simulink model "ofdm_txrx_supermimo_coded"  (Simulink 7.7)
settings: Solver=VariableStepDiscrete  SolverMode=SingleTasking  StartTime=0.0  StopTime=2*simOnly_simLength  RelTol=1e-3
PostLoadFcn:
  | ofdm_rx_supermimo_init
InitFcn:
  | ofdm_rx_supermimo_init
StartFcn:
  | ofdm_rx_supermimo_init
values from neighbouring files (symbols this model uses):
  ActionID_AFTransmit = 31    [ofdm_tx_supermimo_init.m]
  ActionID_Disabled = 0    [ofdm_tx_supermimo_init.m]
  ActionID_SetFlagA = 62    [ofdm_tx_supermimo_init.m]
  ActionID_SetFlagB = 61    [ofdm_tx_supermimo_init.m]
  BER_RAM_init_size = 2048    [ofdm_tx_supermimo_init.m]
  channel_h = [1 0; 1 0] * 0.3    [ofdm_tx_supermimo_init.m]
  CPLength = 16    [ofdm_tx_supermimo_init.m]
  longCorr_coef_i = [-4*real(LTS) 0]    [ofdm_rx_supermimo_init.m]
  longCorr_coef_q = [4*imag(LTS) 0]    [ofdm_rx_supermimo_init.m]
  max_CP_length = 16    [ofdm_tx_supermimo_init.m]
  max_num_baseRateSymbols = 31    [ofdm_tx_supermimo_init.m]
  max_num_subcarriers = 64    [ofdm_tx_supermimo_init.m]
  max_num_trainingSymbols = 15    [ofdm_tx_supermimo_init.m]
  max_numBytes = 16384    [ofdm_tx_supermimo_init.m]
  max_OFDM_symbols = 1023    [ofdm_tx_supermimo_init.m]
  modConstellation_qam16 = .75*[1 3 -1 -3]./3    [ofdm_tx_supermimo_init.m]
  modConstellation_qam64 = 0.875*[3 1 5 7 -3 -1 -5 -7]./7    [ofdm_tx_supermimo_init.m]
  modConstellation_qpsk = [1 -1]./sqrt(2)    [ofdm_tx_supermimo_init.m]
  numBaseRateSymbols = numBaseRateSymbols *2    [ofdm_tx_supermimo_init.m]
  numSubcarriers = 64    [ofdm_tx_supermimo_init.m]
  numTrainingSymbols = 2    [ofdm_tx_supermimo_init.m]
  pktTiming_count_payloadStart = pktTiming_count_lsStart + pktTiming_ls_num*pktTiming_ls_length    [ofdm_rx_supermimo_init.m]
  preamble = preamble_scale*[shortsyms_10 longsyms_2]    [ofdm_tx_supermimo_init.m]
  preamble_ROM = preamble_I_ROM + preamble_Q_ROM*2^16    [ofdm_tx_supermimo_init.m]
  RAM_init_size = max_numBytes    (= 16384)    [ofdm_tx_supermimo_init.m]
  RxDataScrambling_Seq = [40 198 78 63 82 173 102 245 48 111 172 115 147 230 216 93 72 65 62 2 205 242 122 90 128 83 105 97 73 10 5 252]    [ofdm_tx_supermimo_init.m]
  shortSymbol_time = shortSymbol_time(1:16).'    [ofdm_tx_supermimo_init.m]
  simOnly_numSamples = length(preamble)+( (numSubcarriers+CPLength)*(numTrainingSymbols + numBaseRateSymbols + numFullRateSymbols/2) )    [ofdm_tx_supermimo_init.m]
  train = [train train]    [ofdm_tx_supermimo_init.m]
  TxDataScrambling_Seq = [40 198 78 63 82 173 102 245 48 111 172 115 147 230 216 93 72 65 62 2 205 242 122 90 128 83 105 97 73 10 5 252]    [ofdm_tx_supermimo_init.m]
undefined symbols (not defined in the file or in its folder): AntA, AntB, BaseRate, Down, gcbh, numBuffs, numBytes, Up, xlBlockLoadCallback, xlGetNormalizedPeriod, xlipgetpartsetting
libraries: xbsIndex_r4 x5430
(part 18 of 49)

== system ofdm_txrx_supermimo_coded/OFDM Rx MIMO/Equalizer & Packetizer/Packet_Constructor/Slicer ==
  note: 8MSB
  note: 8MSB-7
  SliceSel : Inport
  D : Inport  Port=2
  " byte mux1" : xbsIndex_r4/Mux  inputs=8  en=off  latency=0  precision=Full  arith_type="Signed  (2's comp)"  n_bits=8  bin_pt=2  quantization=Truncate  overflow=Wrap  dbl_ovrd=off  block_type=mux
      settings: xl_area=[16 0 0 32 0 0 0]  block_version=VER_STRING_GOES_HERE
  Slice : xbsIndex_r4/Slice  nbits=8  boolean_output=off  mode=Lower Bit Location + Width  bit1=0  base1=MSB of Input  bit0=0  base0=LSB of Input  dbl_ovrd=off  block_type=slice
      settings: infoedit="Extracts a given range of bits from each input sample and presents it at the output.  The output type is ordinarily unsigned with binary point at zero, but can be Boolean when the slice is one bit wide.<P><P>Hardware notes: In hardware this block costs nothing."  block_version=VER_STRING_GOES_HERE
  Slice1 : xbsIndex_r4/Slice  nbits=8  boolean_output=off  mode=Lower Bit Location + Width  bit1=0  base1=MSB of Input  bit0=1  base0=LSB of Input  dbl_ovrd=off  block_type=slice
      settings: infoedit="Extracts a given range of bits from each input sample and presents it at the output.  The output type is ordinarily unsigned with binary point at zero, but can be Boolean when the slice is one bit wide.<P><P>Hardware notes: In hardware this block costs nothing."  block_version=VER_STRING_GOES_HERE
  Slice2 : xbsIndex_r4/Slice  nbits=8  boolean_output=off  mode=Lower Bit Location + Width  bit1=0  base1=MSB of Input  bit0=2  base0=LSB of Input  dbl_ovrd=off  block_type=slice
      settings: infoedit="Extracts a given range of bits from each input sample and presents it at the output.  The output type is ordinarily unsigned with binary point at zero, but can be Boolean when the slice is one bit wide.<P><P>Hardware notes: In hardware this block costs nothing."  block_version=VER_STRING_GOES_HERE
  Slice3 : xbsIndex_r4/Slice  nbits=8  boolean_output=off  mode=Lower Bit Location + Width  bit1=0  base1=MSB of Input  bit0=3  base0=LSB of Input  dbl_ovrd=off  block_type=slice
      settings: infoedit="Extracts a given range of bits from each input sample and presents it at the output.  The output type is ordinarily unsigned with binary point at zero, but can be Boolean when the slice is one bit wide.<P><P>Hardware notes: In hardware this block costs nothing."  block_version=VER_STRING_GOES_HERE
  Slice4 : xbsIndex_r4/Slice  nbits=8  boolean_output=off  mode=Lower Bit Location + Width  bit1=0  base1=MSB of Input  bit0=4  base0=LSB of Input  dbl_ovrd=off  block_type=slice
      settings: infoedit="Extracts a given range of bits from each input sample and presents it at the output.  The output type is ordinarily unsigned with binary point at zero, but can be Boolean when the slice is one bit wide.<P><P>Hardware notes: In hardware this block costs nothing."  block_version=VER_STRING_GOES_HERE
  Slice5 : xbsIndex_r4/Slice  nbits=8  boolean_output=off  mode=Lower Bit Location + Width  bit1=0  base1=MSB of Input  bit0=5  base0=LSB of Input  dbl_ovrd=off  block_type=slice
      settings: infoedit="Extracts a given range of bits from each input sample and presents it at the output.  The output type is ordinarily unsigned with binary point at zero, but can be Boolean when the slice is one bit wide.<P><P>Hardware notes: In hardware this block costs nothing."  block_version=VER_STRING_GOES_HERE
  Slice6 : xbsIndex_r4/Slice  nbits=8  boolean_output=off  mode=Lower Bit Location + Width  bit1=0  base1=MSB of Input  bit0=6  base0=LSB of Input  dbl_ovrd=off  block_type=slice
      settings: infoedit="Extracts a given range of bits from each input sample and presents it at the output.  The output type is ordinarily unsigned with binary point at zero, but can be Boolean when the slice is one bit wide.<P><P>Hardware notes: In hardware this block costs nothing."  block_version=VER_STRING_GOES_HERE
  Slice7 : xbsIndex_r4/Slice  nbits=8  boolean_output=off  mode=Lower Bit Location + Width  bit1=0  base1=MSB of Input  bit0=7  base0=LSB of Input  dbl_ovrd=off  block_type=slice
      settings: infoedit="Extracts a given range of bits from each input sample and presents it at the output.  The output type is ordinarily unsigned with binary point at zero, but can be Boolean when the slice is one bit wide.<P><P>Hardware notes: In hardware this block costs nothing."  block_version=VER_STRING_GOES_HERE
  Q : Outport
  wires:
  " byte mux1"/1 -> Q/1
  SliceSel/1 -> " byte mux1"/1
  D/1 -> Slice3/1, Slice6/1, Slice7/1, Slice5/1, Slice4/1, Slice2/1, Slice1/1, Slice/1
  Slice/1 -> " byte mux1"/2
  Slice1/1 -> " byte mux1"/3
  Slice2/1 -> " byte mux1"/4
  Slice3/1 -> " byte mux1"/5
  Slice4/1 -> " byte mux1"/6
  Slice5/1 -> " byte mux1"/7
  Slice6/1 -> " byte mux1"/8
  Slice7/1 -> " byte mux1"/9

== system ofdm_txrx_supermimo_coded/OFDM Rx MIMO/Equalizer & Packetizer/Packet_Constructor/Subsystem ==
  In1 : Inport
  In2 : Inport  Port=2
  In3 : Inport  Port=3
  In4 : Inport  Port=4
  In5 : Inport  Port=5
  In6 : Inport  Port=6
  In7 : Inport  Port=7
  In8 : Inport  Port=8
  Simulation Multiplexer{,1..7} (8 alike) : xbsIndex_r4/Simulation Multiplexer  sim_sel=1  hw_sel=2  block_type=simmux
      settings: infoedit="Distinguish input subsystems as \"simulation only\" and \"generation only\".  The input specified For Simulation will be used during Simulink simulation.  The input specified For Generation will be used during code generation.  This block will typically be used on the output of a HW Co-Simulation, Black Box, or ModelSim subsystem.<br><br>Hardware Notes: This block costs nothing."
  Subsystem : Subsystem
  Subsystem1 : Subsystem
  Out1 : Outport
  Out2 : Outport  Port=2
  Out3 : Outport  Port=3
  Out4 : Outport  Port=4
  Out5 : Outport  Port=5
  Out6 : Outport  Port=6
  Out7 : Outport  Port=7
  Out8 : Outport  Port=8
  wires:
  In1/1 -> Subsystem/1(In1), Subsystem1/1(In1)
  In3/1 -> Subsystem/3(In3), Subsystem1/3(In3)
  In4/1 -> Subsystem/4(In4), Subsystem1/4(In4)
  In5/1 -> Subsystem/5(In5), Subsystem1/5(In5)
  In6/1 -> Subsystem/6(In6), Subsystem1/6(In6)
  In7/1 -> Subsystem/7(In7), Subsystem1/7(In7)
  In8/1 -> Subsystem/8(In8), Subsystem1/8(In8)
  In2/1 -> Subsystem/2(In2), Subsystem1/2(In2)
  Simulation Multiplexer/1 -> Out1/1
  Simulation Multiplexer1/1 -> Out2/1
  Simulation Multiplexer2/1 -> Out3/1
  Simulation Multiplexer3/1 -> Out4/1
  Simulation Multiplexer4/1 -> Out5/1
  Simulation Multiplexer5/1 -> Out6/1
  Simulation Multiplexer6/1 -> Out7/1
  Simulation Multiplexer7/1 -> Out8/1
  Subsystem/1(Out1) -> Simulation Multiplexer/1
  Subsystem/2(Out2) -> Simulation Multiplexer1/1
  Subsystem/3(Out3) -> Simulation Multiplexer2/1
  Subsystem/4(Out4) -> Simulation Multiplexer3/1
  Subsystem/5(Out5) -> Simulation Multiplexer4/1
  Subsystem/6(Out6) -> Simulation Multiplexer5/1
  Subsystem/7(Out7) -> Simulation Multiplexer6/1
  Subsystem/8(Out8) -> Simulation Multiplexer7/1
  Subsystem1/1(Out1) -> Simulation Multiplexer/2
  Subsystem1/2(Out2) -> Simulation Multiplexer1/2
  Subsystem1/3(Out3) -> Simulation Multiplexer2/2
  Subsystem1/4(Out4) -> Simulation Multiplexer3/2
  Subsystem1/5(Out5) -> Simulation Multiplexer4/2
  Subsystem1/6(Out6) -> Simulation Multiplexer5/2
  Subsystem1/7(Out7) -> Simulation Multiplexer6/2
  Subsystem1/8(Out8) -> Simulation Multiplexer7/2

== system ofdm_txrx_supermimo_coded/OFDM Rx MIMO/FFT & Chan Est/Addr Gen1/Copy_Downsample ==
  D : Inport
  Delay : xbsIndex_r4/Delay  rst=off  infoeditControl=Selection of Reset will increase slice count due to use of real FFs and instead of SRLs  en=off  latency=1  dbl_ovrd=off  reg_retiming=off  block_type=delay
      settings: infoedit=Hardware notes: A delay line is a chain, each link of which is an SRL16 followed by a flip-flop. If register retiming is enabled, the delay line is a chain of flip-flops.  block_version=VER_STRING_GOES_HERE
  Down Sample : xbsIndex_r4/Down Sample  sample_ratio=2  sample_phase="Last Value of Frame  (most efficient)"  en=off  latency=1  dbl_ovrd=off  block_type=dsamp
      settings: block_version=VER_STRING_GOES_HERE
  Logical : xbsIndex_r4/Logical  logical_function=OR  inputs=2  en=off  latency=0  precision=Full  arith_type=Unsigned  n_bits=16  bin_pt=0  align_bp=on  dbl_ovrd=off  block_type=logical
      settings: block_version=VER_STRING_GOES_HERE
  Q : Outport
  wires:
  Delay/1 -> Logical/1
  D/1 -> Delay/1, Logical/2
  Logical/1 -> Down Sample/1
  Down Sample/1 -> Q/1

== system ofdm_txrx_supermimo_coded/OFDM Rx MIMO/FFT & Chan Est/Addr Gen1/Oscillator ==
  en : Inport
  rst : Inport  Port=2
  Assert : xbsIndex_r4/Assert  assert_type=on  type_source=Explicitly  gui_display_data_type=Fixed-point  arith_type=Boolean  n_bits=16  bin_pt=14  preci_type=Single  exp_width=8  frac_width=24  assert_rate=on  rate_source=Explicitly  period=2  output_port=on  block_type=assert
      settings: infoedit=Asserts a user-defined sample rate and/or type on a signal.<P><P>Hardware notes: In hardware this block costs nothing.  block_version=VER_STRING_GOES_HERE
  Inverter1 : xbsIndex_r4/Inverter  en=off  latency=0  dbl_ovrd=off  block_type=inv
      settings: block_version=VER_STRING_GOES_HERE
  Register : xbsIndex_r4/Register  init=0  rst=on  en=on  dbl_ovrd=off  block_type=register
      settings: block_version=VER_STRING_GOES_HERE
  Q : Outport
  wires:
  Register/1 -> Q/1, Assert/1
  en/1 -> Register/3
  Inverter1/1 -> Register/1
  rst/1 -> Register/2
  Assert/1 -> Inverter1/1

== system ofdm_txrx_supermimo_coded/OFDM Rx MIMO/FFT & Chan Est/Addr Gen1/Output Sym Counter ==
  FFT Done : Inport
  Not Training : Inport  Port=2
  Payload : Inport  Port=3
  Read Addr : Inport  Port=4
  Constant1 : xbsIndex_r4/Constant  const=numSubcarriers-1  gui_display_data_type=Fixed-point  arith_type=Unsigned  n_bits=ceil(log2(numSubcarriers))  bin_pt=0  preci_type=Single  exp_width=8  frac_width=24  explicit_period=off  period=1  equ=P=C  opselect=C  inp2=PCIN>>17  opr=+  inp1=P  carry=CIN  dbl_ovrd=off  block_type=constant
      settings: block_version=VER_STRING_GOES_HERE
  Counter1 : xbsIndex_r4/Counter  cnt_type=Free Running  cnt_to=127  operation=Up  start_count=0  cnt_by_val=1  arith_type=Unsigned  n_bits=ceil(log2(max_OFDM_symbols))  bin_pt=0  load_pin=off  rst=on  en=on  explicit_period=off  period=2  dbl_ovrd=off  use_behavioral_HDL=off  implementation=Fabric  use_rpm=on  block_type=counter
      settings: xl_area=[6 12 0 12 0 0 0]  block_version=VER_STRING_GOES_HERE
  Inverter3 : xbsIndex_r4/Inverter  en=off  latency=0  dbl_ovrd=off  block_type=inv
      settings: block_version=VER_STRING_GOES_HERE
  Logical1 : xbsIndex_r4/Logical  logical_function=AND  inputs=2  en=off  latency=0  precision=Full  arith_type=Unsigned  n_bits=8  bin_pt=2  align_bp=on  dbl_ovrd=off  block_type=logical
      settings: xl_area=[0, 0, 0, 0, 0, 0, 0]  block_version=VER_STRING_GOES_HERE
  Logical2 : xbsIndex_r4/Logical  logical_function=OR  inputs=2  en=off  latency=0  precision=Full  arith_type=Unsigned  n_bits=8  bin_pt=2  align_bp=on  dbl_ovrd=off  block_type=logical
      settings: xl_area=[0, 0, 0, 0, 0, 0, 0]  block_version=VER_STRING_GOES_HERE
  Logical3 : xbsIndex_r4/Logical  logical_function=AND  inputs=2  en=off  latency=0  precision=Full  arith_type=Unsigned  n_bits=8  bin_pt=2  align_bp=on  dbl_ovrd=off  block_type=logical
      settings: xl_area=[0, 0, 0, 0, 0, 0, 0]  block_version=VER_STRING_GOES_HERE
  Logical4 : xbsIndex_r4/Logical  logical_function=AND  inputs=2  en=off  latency=0  precision=Full  arith_type=Unsigned  n_bits=8  bin_pt=2  align_bp=on  dbl_ovrd=off  block_type=logical
      settings: xl_area=[0, 0, 0, 0, 0, 0, 0]  block_version=VER_STRING_GOES_HERE
  Posedge : Subsystem
  Posedge1 : Subsystem
  Posedge2 : Subsystem
  Posedge3 : Subsystem
  Relational : xbsIndex_r4/Relational  mode=a=b  en=off  latency=0  dbl_ovrd=off  block_type=relational
      settings: block_version=VER_STRING_GOES_HERE
  S-R Latch2 : Subsystem
  Sym Index : Outport
  wires:
  Logical4/1 -> Logical3/2
  S-R Latch2/1(D) -> Logical4/2
  Posedge/1(Q) -> S-R Latch2/1(S), Logical4/1
  FFT Done/1 -> Posedge/1(D)
  Counter1/1 -> Sym Index/1
  Not Training/1 -> Inverter3/1, Logical1/2
  Inverter3/1 -> Logical3/1
  Payload/1 -> Posedge1/1(D)
  Posedge1/1(Q) -> S-R Latch2/2(R), Counter1/1
  Read Addr/1 -> Relational/2
  Constant1/1 -> Relational/1
  Relational/1 -> Logical1/1
  Logical1/1 -> Posedge2/1(D)
  Logical3/1 -> Posedge3/1(D)
  Logical2/1 -> Counter1/2
  Posedge2/1(Q) -> Logical2/1
  Posedge3/1(Q) -> Logical2/2

== system ofdm_txrx_supermimo_coded/OFDM Rx MIMO/FFT & Chan Est/Addr Gen1/Rd Delay ==
  note: Delay the equalizer inputs in Alamout mode in order
      | to calculate pilot phaes for both r0 and r1 before
      | starting the decoding process. In SISO/Multiplexing mode,
      | each symbol has its own pilots, so there's a shorter delay.
  D : Inport
  Delay1 : xbsIndex_r4/Delay  rst=off  infoeditControl=Selection of Reset will increase slice count due to use of real FFs and instead of SRLs  en=off  latency=3  dbl_ovrd=off  reg_retiming=off  block_type=delay
      settings: block_version=10.1.2
  Delay2 : xbsIndex_r4/Delay  rst=off  infoeditControl=Selection of Reset will increase slice count due to use of real FFs and instead of SRLs  en=off  latency=35  dbl_ovrd=off  reg_retiming=off  block_type=delay
      settings: block_version=10.1.2
  Inverter1 : xbsIndex_r4/Inverter  en=off  latency=0  dbl_ovrd=off  block_type=inv
  Logical1 : xbsIndex_r4/Logical  logical_function=AND  inputs=2  en=off  latency=0  precision=Full  arith_type=Unsigned  n_bits=16  bin_pt=0  align_bp=on  dbl_ovrd=off  block_type=logical
  Logical2 : xbsIndex_r4/Logical  logical_function=OR  inputs=2  en=off  latency=0  precision=Full  arith_type=Unsigned  n_bits=16  bin_pt=0  align_bp=on  dbl_ovrd=off  block_type=logical
  Logical3 : xbsIndex_r4/Logical  logical_function=AND  inputs=2  en=off  latency=0  precision=Full  arith_type=Unsigned  n_bits=16  bin_pt=0  align_bp=on  dbl_ovrd=off  block_type=logical
  Q : Outport
  wires:
  Logical1/1 -> Logical2/1
  Logical3/1 -> Logical2/2
  Logical2/1 -> Q/1
  [regRx_AlamoutiMode] -> Logical1/2, Inverter1/1
  Inverter1/1 -> Logical3/1
  Delay2/1 -> Logical1/1
  D/1 -> Delay2/1, Delay1/1
  Delay1/1 -> Logical3/2

== system ofdm_txrx_supermimo_coded/OFDM Rx MIMO/FFT & Chan Est/Addr Gen1/S-R Latch1 ==
  S : Inport
  R : Inport  Port=2
  Constant2 : xbsIndex_r4/Constant  const=1  gui_display_data_type=Fixed-point  arith_type=Boolean  n_bits=1  bin_pt=0  preci_type=Single  exp_width=8  frac_width=24  explicit_period=off  period=1  equ=P=C  opselect=C  inp2=PCIN>>17  opr=+  inp1=P  carry=CIN  dbl_ovrd=off  block_type=constant
      settings: block_version=VER_STRING_GOES_HERE
  Register : xbsIndex_r4/Register  init=0  rst=on  en=on  dbl_ovrd=off  block_type=register
      settings: xl_area=[0, 0, 0, 0, 0, 0, 0]  block_version=VER_STRING_GOES_HERE
  D : Outport
  wires:
  Register/1 -> D/1
  S/1 -> Register/3
  R/1 -> Register/2
  Constant2/1 -> Register/1

== system ofdm_txrx_supermimo_coded/OFDM Rx MIMO/FFT & Chan Est/Addr_Generator/Initial Read Addr Timing Calc ==
  note: The FFT runs 4x as fast as the input samples. As the input
      | samples are written to the buffers, the FFT waits to start
      | until it can have access to the newest sample it needs
      | 1 cycle after it's written.
  AddSub1 : xbsIndex_r4/AddSub  mode=Addition  use_carryin=off  use_carryout=off  en=off  latency=0  precision=User Defined  arith_type=Unsigned  n_bits=ceil(log2(max_num_subcarriers))+1  bin_pt=0  quantization=Truncate  overflow=Wrap  dbl_ovrd=off  use_behavioral_HDL=on  hw_selection=Fabric  pipelined=off  use_rpm=on  block_type=addsub
      settings: xl_area=[4 0 0 6 0 0 0]  block_version=VER_STRING_GOES_HERE
  Constant1 : xbsIndex_r4/Constant  const=0.75*numSubcarriers  gui_display_data_type=Fixed-point  arith_type=Unsigned  n_bits=log2(numSubcarriers)  bin_pt=0  preci_type=Single  exp_width=8  frac_width=24  explicit_period=off  period=1  equ=P=C  opselect=C  inp2=PCIN>>17  opr=+  inp1=P  carry=CIN  dbl_ovrd=off  block_type=constant
      settings: block_version=VER_STRING_GOES_HERE
  Up Sample4 : xbsIndex_r4/Up Sample  sample_ratio=2  copy_samples=on  en=off  latency=0  dbl_ovrd=off  block_type=usamp
      settings: infoedit="Up samples input data.  Inserted values  can be zeros or copies of the most recent input sample.<P><P>Hardware notes: No hardware is needed if inserted values are copies of the input sample; otherwise, a mux and single bit flip-flop are used."  block_version=VER_STRING_GOES_HERE
  D : Outport
  wires:
  AddSub1/1 -> D/1
  [regRx_symbolTimingOffset] -> Up Sample4/1
  Up Sample4/1 -> AddSub1/2
  Constant1/1 -> AddSub1/1

== system ofdm_txrx_supermimo_coded/OFDM Rx MIMO/FFT & Chan Est/Addr_Generator/Oscillator ==
  en : Inport
  rst : Inport  Port=2
  Assert : xbsIndex_r4/Assert  assert_type=on  type_source=Explicitly  gui_display_data_type=Fixed-point  arith_type=Boolean  n_bits=16  bin_pt=14  preci_type=Single  exp_width=8  frac_width=24  assert_rate=on  rate_source=Explicitly  period=4  output_port=on  block_type=assert
      settings: infoedit=Asserts a user-defined sample rate and/or type on a signal.<P><P>Hardware notes: In hardware this block costs nothing.  block_version=VER_STRING_GOES_HERE
  Inverter1 : xbsIndex_r4/Inverter  en=off  latency=0  dbl_ovrd=off  block_type=inv
      settings: block_version=VER_STRING_GOES_HERE
  Register1 : xbsIndex_r4/Register  init=0  rst=on  en=on  dbl_ovrd=off  block_type=register
      settings: block_version=VER_STRING_GOES_HERE
  Q : Outport
  wires:
  rst/1 -> Register1/2
  Assert/1 -> Inverter1/1
  Inverter1/1 -> Register1/1
  en/1 -> Register1/3
  Register1/1 -> Q/1, Assert/1

== system ofdm_txrx_supermimo_coded/OFDM Rx MIMO/FFT & Chan Est/Addr_Generator/Posedge ==
  D : Inport
  Delay : xbsIndex_r4/Delay  rst=off  infoeditControl=Selection of Reset will increase slice count due to use of real FFs and instead of SRLs  en=off  latency=1  dbl_ovrd=off  reg_retiming=off  block_type=delay
      settings: infoedit=Hardware notes: A delay line is a chain, each link of which is an SRL16 followed by a flip-flop. If register retiming is enabled, the delay line is a chain of flip-flops.  xl_area=[1 1 0 0 0 0 0]  block_version=VER_STRING_GOES_HERE
  Inverter : xbsIndex_r4/Inverter  en=off  latency=0  dbl_ovrd=off  block_type=inv
      settings: xl_area=[0 0 0 0 0 0 0]  block_version=VER_STRING_GOES_HERE
  Logical : xbsIndex_r4/Logical  logical_function=AND  inputs=2  en=off  latency=0  precision=Full  arith_type=Unsigned  n_bits=8  bin_pt=2  align_bp=on  dbl_ovrd=off  block_type=logical
      settings: xl_area=[1 0 0 1 0 0 0]  block_version=VER_STRING_GOES_HERE
  Q : Outport
  wires:
  Logical/1 -> Q/1
  D/1 -> Delay/1, Logical/1
  Inverter/1 -> Logical/2
  Delay/1 -> Inverter/1

== system ofdm_txrx_supermimo_coded/OFDM Rx MIMO/FFT & Chan Est/Addr_Generator/negedge ==
  D : Inport
  Delay : xbsIndex_r4/Delay  rst=off  infoeditControl=Selection of Reset will increase slice count due to use of real FFs and instead of SRLs  en=off  latency=1  dbl_ovrd=off  reg_retiming=off  block_type=delay
      settings: infoedit=Hardware notes: A delay line is a chain, each link of which is an SRL16 followed by a flip-flop. If register retiming is enabled, the delay line is a chain of flip-flops.  xl_area=[1 1 0 0 0 0 0]  block_version=VER_STRING_GOES_HERE
  Inverter : xbsIndex_r4/Inverter  en=off  latency=0  dbl_ovrd=off  block_type=inv
      settings: xl_area=[0 0 0 0 0 0 0]  block_version=VER_STRING_GOES_HERE
  Logical : xbsIndex_r4/Logical  logical_function=AND  inputs=2  en=off  latency=0  precision=Full  arith_type=Unsigned  n_bits=8  bin_pt=2  align_bp=on  dbl_ovrd=off  block_type=logical
      settings: xl_area=[1 0 0 1 0 0 0]  block_version=VER_STRING_GOES_HERE
  Q : Outport
  wires:
  Inverter/1 -> Logical/2
  D/1 -> Delay/1, Inverter/1
  Logical/1 -> Q/1
  Delay/1 -> Logical/1

== system ofdm_txrx_supermimo_coded/OFDM Rx MIMO/FFT & Chan Est/Addr_Generator/negedge1 ==
  D : Inport
  Delay : xbsIndex_r4/Delay  rst=off  infoeditControl=Selection of Reset will increase slice count due to use of real FFs and instead of SRLs  en=off  latency=1  dbl_ovrd=off  reg_retiming=off  block_type=delay
      settings: infoedit=Hardware notes: A delay line is a chain, each link of which is an SRL16 followed by a flip-flop. If register retiming is enabled, the delay line is a chain of flip-flops.  xl_area=[1 1 0 0 0 0 0]  block_version=VER_STRING_GOES_HERE
  Inverter : xbsIndex_r4/Inverter  en=off  latency=0  dbl_ovrd=off  block_type=inv
      settings: xl_area=[0 0 0 0 0 0 0]  block_version=VER_STRING_GOES_HERE
  Logical : xbsIndex_r4/Logical  logical_function=AND  inputs=2  en=off  latency=0  precision=Full  arith_type=Unsigned  n_bits=8  bin_pt=2  align_bp=on  dbl_ovrd=off  block_type=logical
      settings: xl_area=[1 0 0 1 0 0 0]  block_version=VER_STRING_GOES_HERE
  Q : Outport
  wires:
  Inverter/1 -> Logical/1
  Delay/1 -> Logical/2
  D/1 -> Inverter/1, Delay/1
  Logical/1 -> Q/1

== system ofdm_txrx_supermimo_coded/OFDM Rx MIMO/FFT & Chan Est/Channel Estimation & Pilot Processing/Actual Trainig Window ==
  H Vin : Inport
  Train Sym : Inport  Port=2
  Logical2 : xbsIndex_r4/Logical  logical_function=AND  inputs=2  en=off  latency=0  precision=Full  arith_type=Unsigned  n_bits=8  bin_pt=2  align_bp=on  dbl_ovrd=off  block_type=logical
      settings: xl_area=[1 0 0 1 0 0 0]  block_version=VER_STRING_GOES_HERE
  Posedge : Subsystem
  S-R Latch : Subsystem
  negedge : Subsystem
  Train : Outport
  wires:
  S-R Latch/1(D) -> Logical2/1
  Posedge/1(Q) -> S-R Latch/1(S)
  negedge/1(Q) -> S-R Latch/2(R)
  H Vin/1 -> Posedge/1(D)
  Logical2/1 -> Train/1
  Train Sym/1 -> negedge/1(D), Logical2/2

== system ofdm_txrx_supermimo_coded/OFDM Rx MIMO/FFT & Chan Est/Channel Estimation & Pilot Processing/Bus Creator ==
  AA-I : Inport
  AA-Q : Inport  Port=2
  AB-I : Inport  Port=3
  AB-Q : Inport  Port=4
  BA-I : Inport  Port=5
  BA-Q : Inport  Port=6
  BB-I : Inport  Port=7
  BB-Q : Inport  Port=8
  Bus Creator1 : BusCreator
  Bus Creator2 : BusCreator
  Constant1 : xbsIndex_r4/Constant  const=0  gui_display_data_type=Fixed-point  arith_type=Signed (2's comp)  n_bits=18  bin_pt=16  preci_type=Single  exp_width=8  frac_width=24  explicit_period=on  period=2  equ=P=C  opselect=C  inp2=PCIN>>17  opr=+  inp1=P  carry=CIN  dbl_ovrd=off  block_type=constant
      settings: block_version=VER_STRING_GOES_HERE
  Constant2 : xbsIndex_r4/Constant  const=0  gui_display_data_type=Fixed-point  arith_type=Signed (2's comp)  n_bits=18  bin_pt=16  preci_type=Single  exp_width=8  frac_width=24  explicit_period=on  period=2  equ=P=C  opselect=C  inp2=PCIN>>17  opr=+  inp1=P  carry=CIN  dbl_ovrd=off  block_type=constant
      settings: block_version=VER_STRING_GOES_HERE
  I : Outport
  Q : Outport  Port=2
  wires:
  AA-I/1 -> Bus Creator1/1
  AB-I/1 -> Bus Creator1/2
  Bus Creator1/1 -> I/1
  BA-I/1 -> Bus Creator1/3
  BB-I/1 -> Bus Creator1/4
  AA-Q/1 -> Bus Creator2/1
  AB-Q/1 -> Bus Creator2/2
  Bus Creator2/1 -> Q/1
  BA-Q/1 -> Bus Creator2/3
  BB-Q/1 -> Bus Creator2/4

== system ofdm_txrx_supermimo_coded/OFDM Rx MIMO/FFT & Chan Est/Channel Estimation & Pilot Processing/Chan Est Buffer & Mag Calc ==
  note: Stores each channel coefficient as a
      | 32-bit word, combining [I Q] per word.
  AA-I : Inport
  AA-Q : Inport  Port=2
  AB-I : Inport  Port=3
  AB-Q : Inport  Port=4
  BA-I : Inport  Port=5
  BA-Q : Inport  Port=6
  BB-I : Inport  Port=7
  BB-Q : Inport  Port=8
  Est Vin : Inport  Port=9
  SubCar Index : Inport  Port=10
  1MSB : xbsIndex_r4/Slice  nbits=1  boolean_output=on  mode=Upper Bit Location + Width  bit1=0  base1=MSB of Input  bit0=0  base0=LSB of Input  dbl_ovrd=off  block_type=slice
      settings: infoedit="Extracts a given range of bits from each input sample and presents it at the output.  The output type is ordinarily unsigned with binary point at zero, but can be Boolean when the slice is one bit wide.<P><P>Hardware notes: In hardware this block costs nothing."  block_version=VER_STRING_GOES_HERE
  "2x16b -> 32b" : Subsystem
  Concat : xbsIndex_r4/Concat  num_inputs=2  dbl_ovrd=off  block_type=concat
      settings: block_version=10.1.3
  Convert{3..10} (8 alike) : xbsIndex_r4/Convert  gui_display_data_type=Fixed-point  arith_type="Signed  (2's comp)"  n_bits=16  bin_pt=14  float_type=Single  exp_bits=8  fraction_bits=24  quantization=Truncate  overflow=Flag as error  en=off  latency=0  dbl_ovrd=off  pipeline=off  block_type=convert
      settings: xl_area=[0, 0, 0, 0, 0, 0, 0]  block_version=VER_STRING_GOES_HERE
  Counter : xbsIndex_r4/Counter  cnt_type=Free Running  cnt_to=Inf  operation=Up  start_count=0  cnt_by_val=1  arith_type=Unsigned  n_bits=2  bin_pt=0  load_pin=off  rst=on  en=on  explicit_period=off  period=1  dbl_ovrd=off  use_behavioral_HDL=off  implementation=Fabric  use_rpm=on  block_type=counter
      settings: xl_area=[6 12 0 12 0 0 0]  block_version=VER_STRING_GOES_HERE
  Delay{1..5} (5 alike) : xbsIndex_r4/Delay  rst=off  infoeditControl=Selection of Reset will increase slice count due to use of real FFs and instead of SRLs  en=off  latency=1  dbl_ovrd=off  reg_retiming=off  block_type=delay
      settings: infoedit=Hardware notes: A delay line is a chain, each link of which is an SRL16 followed by a flip-flop. If register retiming is enabled, the delay line is a chain of flip-flops.  xl_area=[0, 0, 0, 0, 0, 0, 0]  block_version=VER_STRING_GOES_HERE
  Logical1 : xbsIndex_r4/Logical  logical_function=AND  inputs=2  en=off  latency=0  precision=Full  arith_type=Unsigned  n_bits=8  bin_pt=2  align_bp=on  dbl_ovrd=off  block_type=logical
      settings: xl_area=[1 0 0 1 0 0 0]  block_version=VER_STRING_GOES_HERE
  Logical2 : xbsIndex_r4/Logical  logical_function=AND  inputs=2  en=off  latency=0  precision=Full  arith_type=Unsigned  n_bits=8  bin_pt=2  align_bp=on  dbl_ovrd=off  block_type=logical
      settings: xl_area=[1 0 0 1 0 0 0]  block_version=VER_STRING_GOES_HERE
  Mux1 : xbsIndex_r4/Mux  inputs=4  en=off  latency=1  precision=Full  arith_type="Signed  (2's comp)"  n_bits=8  bin_pt=2  quantization=Truncate  overflow=Wrap  dbl_ovrd=off  block_type=mux
      settings: xl_area=[0, 0, 0, 0, 0, 0, 0]  block_version=VER_STRING_GOES_HERE
  Mux2 : xbsIndex_r4/Mux  inputs=4  en=off  latency=1  precision=Full  arith_type="Signed  (2's comp)"  n_bits=8  bin_pt=2  quantization=Truncate  overflow=Wrap  dbl_ovrd=off  block_type=mux
      settings: xl_area=[0, 0, 0, 0, 0, 0, 0]  block_version=VER_STRING_GOES_HERE
  Negedge : Subsystem
  Scope : Scope  NumInputPorts=6
      settings: YMin=-5~-5~-5~-5~-5~-5  YMax=5~5~5~5~5~5  SaveName=ScopeData6
  Shared Memory : xbsIndex_r4/Shared Memory  shared_memory_name='ChannelEstimates'  depth=numSubcarriers * 4  ownership=Locally Owned and Initialized  initVector=zeros(1,numSubcarriers*4)  en=off  mutex=Unprotected  mode=Read and Write  write_mode=Read After Write  time_out=0  latency=1  explicit_data_type=on  gui_display_data_type=Fixed-point  arith_type=Unsigned  n_bits=32  bin_pt=0  preci_type=Single  implementation=Block RAM  use_rpm=off  block_type=shmem
      settings: AttributesFormatString=<< %<shared_memory_name> >>  block_version=10.1.3
  done{1..5,9} (6 alike) : xbsIndex_r4/Gateway Out  inherit_from_input=off  hdl_port=off  timing_constraint=None  locs_specified=off  LOCs={}  UseAsDAC=off  DACChannel='1'  block_type=gatewayout
      settings: infoedit="Gateway out block.  Converts Xilinx fixed point inputs into ouputs of type Simulink integer, double, or fixed point.<P><P>Hardware notes:  In hardware these blocks become top level output ports or are discarded, depending on how they are configured."  xl_area=[0 0 0 0 1 0 0]  block_version=VER_STRING_GOES_HERE
  edge : Subsystem
  wires:
  SubCar Index/1 -> Delay1/1, 1MSB/1, done1/1
  Est Vin/1 -> edge/1(D), Logical1/1, Logical2/2
  1MSB/1 -> Negedge/1(D)
  Negedge/1(Q) -> Logical1/2
  Logical1/1 -> Counter/2
  edge/1(Q) -> Counter/1
  "2x16b -> 32b"/1("[I Q]") -> Shared Memory/2
  Concat/1 -> Delay2/1
  Counter/1 -> Mux2/1, Mux1/1, done5/1, Concat/1
  AA-I/1 -> Convert3/1
  AB-I/1 -> Convert4/1
  BA-I/1 -> Convert5/1
  BB-I/1 -> Convert6/1
  AA-Q/1 -> Convert7/1
  AB-Q/1 -> Convert8/1
  BA-Q/1 -> Convert9/1
  BB-Q/1 -> Convert10/1
  Convert7/1 -> Mux1/2
  Convert8/1 -> Mux1/3
  Convert9/1 -> Mux1/4
  Convert10/1 -> Mux1/5
  Convert3/1 -> Mux2/2
  Convert4/1 -> Mux2/3
  Convert5/1 -> Mux2/4
  Convert6/1 -> Mux2/5
  Mux2/1 -> "2x16b -> 32b"/1(I), done9/1
  Delay1/1 -> Concat/2
  Mux1/1 -> "2x16b -> 32b"/2(Q)
  Delay2/1 -> Delay3/1
  Delay3/1 -> Shared Memory/1, done2/1
  done9/1 -> Scope/1
  done1/1 -> Scope/2
  done2/1 -> Scope/3
  done3/1 -> Scope/4
  done4/1 -> Scope/5
  done5/1 -> Scope/6
  Logical2/1 -> Delay5/1
  [regRx_chanEst_RecordEn] -> Logical2/1
  Delay4/1 -> Shared Memory/3, done4/1
  Delay5/1 -> Delay4/1
  "2x16b -> 32b"/2(H_Mag) -> done3/1

== system ofdm_txrx_supermimo_coded/OFDM Rx MIMO/FFT & Chan Est/Channel Estimation & Pilot Processing/Downsamplng ==
  AA-I : Inport
  AA-Q : Inport  Port=2
  AB-I : Inport  Port=3
  AB-Q : Inport  Port=4
  BA-I : Inport  Port=5
  BA-Q : Inport  Port=6
  BB-I : Inport  Port=7
  BB-Q : Inport  Port=8
  Down Sample{,1..7} (8 alike) : xbsIndex_r4/Down Sample  sample_ratio=2  sample_phase="Last Value of Frame  (most efficient)"  en=off  latency=1  dbl_ovrd=off  block_type=dsamp
      settings: block_version=10.1.2
  " AA-I" : Outport
  " AA-Q" : Outport  Port=2
  " AB-I" : Outport  Port=3
  " AB-Q" : Outport  Port=4
  " BA-I" : Outport  Port=5
  " BA-Q" : Outport  Port=6
  " BB-I" : Outport  Port=7
  " BB-Q" : Outport  Port=8
  wires:
  AA-I/1 -> Down Sample/1
  AB-I/1 -> Down Sample1/1
  AA-Q/1 -> Down Sample4/1
  BA-I/1 -> Down Sample2/1
  AB-Q/1 -> Down Sample5/1
  BB-I/1 -> Down Sample3/1
  BA-Q/1 -> Down Sample6/1
  BB-Q/1 -> Down Sample7/1
  Down Sample/1 -> " AA-I"/1
  Down Sample1/1 -> " AB-I"/1
  Down Sample2/1 -> " BA-I"/1
  Down Sample3/1 -> " BB-I"/1
  Down Sample4/1 -> " AA-Q"/1
  Down Sample5/1 -> " AB-Q"/1
  Down Sample6/1 -> " BA-Q"/1
  Down Sample7/1 -> " BB-Q"/1

== system ofdm_txrx_supermimo_coded/OFDM Rx MIMO/FFT & Chan Est/Channel Estimation & Pilot Processing/H_A-A Estimate Buffer ==
  I : Inport
  Q : Inport  Port=2
  Est_WE : Inport  Port=3
  Est_Reset : Inport  Port=4
  FFT_Addr : Inport  Port=5
  EQ_Addr : Inport  Port=6
  I Estimate Buffer : Subsystem
  Q Estimate Buffer : Subsystem
  EstI_fast : Outport
  EstQ_fast : Outport  Port=2
  EstI : Outport  Port=3
  EstQ : Outport  Port=4
  wires:
  Est_Reset/1 -> I Estimate Buffer/4(Rst), Q Estimate Buffer/4(Rst)
  Est_WE/1 -> I Estimate Buffer/3(Est_WE), Q Estimate Buffer/3(Est_WE)
  I Estimate Buffer/1(h[n]) -> EstI/1
  FFT_Addr/1 -> I Estimate Buffer/2(Fast_Addr), Q Estimate Buffer/2(Fast_Addr)
  I/1 -> I Estimate Buffer/1(Est_Data)
  I Estimate Buffer/2(h[n] (fast)) -> EstI_fast/1
  EQ_Addr/1 -> I Estimate Buffer/5(Slow_Addr), Q Estimate Buffer/5(Slow_Addr)
  Q/1 -> Q Estimate Buffer/1(Est_Data)
  Q Estimate Buffer/1(h[n]) -> EstQ/1
  Q Estimate Buffer/2(h[n] (fast)) -> EstQ_fast/1

== system ofdm_txrx_supermimo_coded/OFDM Rx MIMO/FFT & Chan Est/Channel Estimation & Pilot Processing/H_A-B Estimate Buffer ==
  I : Inport
  Q : Inport  Port=2
  Est_WE : Inport  Port=3
  Est_Reset : Inport  Port=4
  Xk_Index : Inport  Port=5
  I Estimate Buffer : Subsystem
  Q Estimate Buffer : Subsystem
  EstI : Outport
  EstQ : Outport  Port=2
  wires:
  I/1 -> I Estimate Buffer/1(Est_Data)
  Xk_Index/1 -> I Estimate Buffer/2(Est_Addr), Q Estimate Buffer/2(Est_Addr)
  I Estimate Buffer/1(h[n]) -> EstI/1
  Est_WE/1 -> I Estimate Buffer/3(Est_WE), Q Estimate Buffer/3(Est_WE)
  Est_Reset/1 -> I Estimate Buffer/4(Rst), Q Estimate Buffer/4(Rst)
  Q Estimate Buffer/1(h[n]) -> EstQ/1
  Q/1 -> Q Estimate Buffer/1(Est_Data)

== system ofdm_txrx_supermimo_coded/OFDM Rx MIMO/FFT & Chan Est/Channel Estimation & Pilot Processing/H_B-A Estimate Buffer ==
  I : Inport
  Q : Inport  Port=2
  Est_WE : Inport  Port=3
  Est_Reset : Inport  Port=4
  FFT_Addr : Inport  Port=5
  EQ_Addr : Inport  Port=6
  I Estimate Buffer : Subsystem
  Q Estimate Buffer : Subsystem
  EstI_fast : Outport
  EstQ_fast : Outport  Port=2
  EstI : Outport  Port=3
  EstQ : Outport  Port=4
  wires:
  Q Estimate Buffer/2(h[n] (fast)) -> EstQ_fast/1
  Q Estimate Buffer/1(h[n]) -> EstQ/1
  Q/1 -> Q Estimate Buffer/1(Est_Data)
  EQ_Addr/1 -> Q Estimate Buffer/5(Slow_Addr), I Estimate Buffer/5(Slow_Addr)
  I Estimate Buffer/2(h[n] (fast)) -> EstI_fast/1
  I/1 -> I Estimate Buffer/1(Est_Data)
  FFT_Addr/1 -> Q Estimate Buffer/2(Fast_Addr), I Estimate Buffer/2(Fast_Addr)
  I Estimate Buffer/1(h[n]) -> EstI/1
  Est_WE/1 -> Q Estimate Buffer/3(Est_WE), I Estimate Buffer/3(Est_WE)
  Est_Reset/1 -> Q Estimate Buffer/4(Rst), I Estimate Buffer/4(Rst)

== system ofdm_txrx_supermimo_coded/OFDM Rx MIMO/FFT & Chan Est/Channel Estimation & Pilot Processing/H_B-B Estimate Buffer ==
  I : Inport
  Q : Inport  Port=2
  Est_WE : Inport  Port=3
  Est_Reset : Inport  Port=4
  Xk_Index : Inport  Port=5
  I Estimate Buffer : Subsystem
  Q Estimate Buffer : Subsystem
  EstI : Outport
  EstQ : Outport  Port=2
  wires:
  Q/1 -> Q Estimate Buffer/1(Est_Data)
  Q Estimate Buffer/1(h[n]) -> EstQ/1
  Est_Reset/1 -> Q Estimate Buffer/4(Rst), I Estimate Buffer/4(Rst)
  Est_WE/1 -> Q Estimate Buffer/3(Est_WE), I Estimate Buffer/3(Est_WE)
  I Estimate Buffer/1(h[n]) -> EstI/1
  Xk_Index/1 -> Q Estimate Buffer/2(Est_Addr), I Estimate Buffer/2(Est_Addr)
  I/1 -> I Estimate Buffer/1(Est_Data)

== system ofdm_txrx_supermimo_coded/OFDM Rx MIMO/FFT & Chan Est/Channel Estimation & Pilot Processing/Mult by training ==
  AR : Inport
  AI : Inport  Port=2
  BR : Inport  Port=3
  Constant1 : xbsIndex_r4/Constant  const=0  gui_display_data_type=Fixed-point  arith_type=Signed (2's comp)  n_bits=2  bin_pt=1  preci_type=Single  exp_width=8  frac_width=24  explicit_period=off  period=1  equ=P=C  opselect=C  inp2=PCIN>>17  opr=+  inp1=P  carry=CIN  dbl_ovrd=off  block_type=constant
      settings: block_version=VER_STRING_GOES_HERE
  Constant2 : xbsIndex_r4/Constant  const=0  gui_display_data_type=Fixed-point  arith_type=Signed (2's comp)  n_bits=2  bin_pt=1  preci_type=Single  exp_width=8  frac_width=24  explicit_period=off  period=1  equ=P=C  opselect=C  inp2=PCIN>>17  opr=+  inp1=P  carry=CIN  dbl_ovrd=off  block_type=constant
      settings: block_version=VER_STRING_GOES_HERE
  Mux3 : xbsIndex_r4/Mux  inputs=3  en=off  latency=1  precision=Full  arith_type="Signed  (2's comp)"  n_bits=8  bin_pt=2  quantization=Truncate  overflow=Wrap  dbl_ovrd=off  block_type=mux
      settings: xl_area=[0, 0, 0, 0, 0, 0, 0]  block_version=VER_STRING_GOES_HERE
  Mux4 : xbsIndex_r4/Mux  inputs=3  en=off  latency=1  precision=Full  arith_type="Signed  (2's comp)"  n_bits=8  bin_pt=2  quantization=Truncate  overflow=Wrap  dbl_ovrd=off  block_type=mux
      settings: xl_area=[0, 0, 0, 0, 0, 0, 0]  block_version=VER_STRING_GOES_HERE
  Negate : xbsIndex_r4/Negate  precision=User Defined  arith_type="Signed  (2's comp)"  n_bits=16  bin_pt=15  quantization=Truncate  overflow=Flag as error  en=off  latency=0  dbl_ovrd=off  block_type=negate
      settings: xl_area=[0, 0, 0, 0, 0, 0, 0]  block_version=VER_STRING_GOES_HERE
  Negate1 : xbsIndex_r4/Negate  precision=User Defined  arith_type="Signed  (2's comp)"  n_bits=16  bin_pt=15  quantization=Truncate  overflow=Flag as error  en=off  latency=0  dbl_ovrd=off  block_type=negate
      settings: xl_area=[0, 0, 0, 0, 0, 0, 0]  block_version=VER_STRING_GOES_HERE
  Reinterpret : xbsIndex_r4/Reinterpret  force_arith_type=on  arith_type=Unsigned  force_bin_pt=on  bin_pt=0  block_type=cast
      settings: infoedit="Changes type of samples without altering their binary representation.<P><P>Hardware notes: In hardware this block costs nothing.<P><P>Example:  Suppose the input is 6 bits wide, signed, with 2 fractional bits, and the output is forced to unsigned with 0 fractional bits.  Then an input of -2.0 (1110.00 in binary 2's complement) becomes an output of 56 (111000 in binary)."  block_version=VER_STRING_GOES_HERE
  PR : Outport
  PI : Outport  Port=2
  wires:
  Reinterpret/1 -> Mux4/1, Mux3/1
  Mux3/1 -> PR/1
  Negate/1 -> Mux3/4
  Constant2/1 -> Mux3/2
  Mux4/1 -> PI/1
  Negate1/1 -> Mux4/4
  Constant1/1 -> Mux4/2
  AI/1 -> Negate1/1, Mux4/3
  BR/1 -> Reinterpret/1
  AR/1 -> Mux3/3, Negate/1

== system ofdm_txrx_supermimo_coded/OFDM Rx MIMO/FFT & Chan Est/Channel Estimation & Pilot Processing/Pilot Processing ==
  Rst : Inport
  I : Inport  Port=2
  Q : Inport  Port=3
  Xk_Index : Inport  Port=4
  Sym_Index : Inport  Port=5
  Train : Inport  Port=6
  HAA_I : Inport  Port=7
  HAA_Q : Inport  Port=8
  HBA_I : Inport  Port=9
  HBA_Q : Inport  Port=10
  CFO Calculation : Subsystem
  Convert3 : xbsIndex_r4/Convert  gui_display_data_type=Fixed-point  arith_type="Signed  (2's comp)"  n_bits=14  bin_pt=13  float_type=Single  exp_bits=8  fraction_bits=24  quantization=Truncate  overflow=Wrap  en=off  latency=0  dbl_ovrd=off  pipeline=off  block_type=convert
      settings: xl_area=[0, 0, 0, 0, 0, 0, 0]  block_version=VER_STRING_GOES_HERE
  Delay12 : xbsIndex_r4/Delay  rst=off  infoeditControl=Selection of Reset will increase slice count due to use of real FFs and instead of SRLs  en=off  latency=2  dbl_ovrd=off  reg_retiming=off  block_type=delay
      settings: infoedit=Hardware notes: A delay line is a chain, each link of which is an SRL16 followed by a flip-flop. If register retiming is enabled, the delay line is a chain of flip-flops.  xl_area=[0, 0, 0, 0, 0, 0, 0]  block_version=VER_STRING_GOES_HERE
  Delay13 : xbsIndex_r4/Delay  rst=off  infoeditControl=Selection of Reset will increase slice count due to use of real FFs and instead of SRLs  en=off  latency=2  dbl_ovrd=off  reg_retiming=off  block_type=delay
      settings: infoedit=Hardware notes: A delay line is a chain, each link of which is an SRL16 followed by a flip-flop. If register retiming is enabled, the delay line is a chain of flip-flops.  xl_area=[0, 0, 0, 0, 0, 0, 0]  block_version=VER_STRING_GOES_HERE
  Delay14 : xbsIndex_r4/Delay  rst=off  infoeditControl=Selection of Reset will increase slice count due to use of real FFs and instead of SRLs  en=off  latency=1  dbl_ovrd=off  reg_retiming=off  block_type=delay
      settings: infoedit=Hardware notes: A delay line is a chain, each link of which is an SRL16 followed by a flip-flop. If register retiming is enabled, the delay line is a chain of flip-flops.  xl_area=[0, 0, 0, 0, 0, 0, 0]  block_version=VER_STRING_GOES_HERE
  Phase Noise Tracker : Subsystem
  Pilot Phase Error Calc : Subsystem
  Register2 : xbsIndex_r4/Register  init=0  rst=on  en=on  dbl_ovrd=off  block_type=register
      settings: block_version=8.2.02
  Reinterpret : xbsIndex_r4/Reinterpret  force_arith_type=on  arith_type=Unsigned  force_bin_pt=on  bin_pt=0  block_type=reinterpret
      settings: infoedit="Changes signal type without altering the binary representation.   You can changed the signal between signed and unsigned, and relocate the binary point.<P><P>Hardware notes: In hardware this block costs nothing.<P><P>Example:  Suppose the input is 6 bits wide, signed, with 2 fractional bits, and the output is forced to unsigned with 0 fractional bits.  Then an input of -2.0 (1110.00 in binary 2's complement) becomes an output of 56 (111000 in binary)."  block_version=10.1
  Rx_debug_PhaseError : xbsIndex_r4/Gateway Out  inherit_from_input=off  hdl_port=on  timing_constraint=None  locs_specified=off  LOCs={}  UseAsDAC=off  DACChannel='1'  block_type=gatewayout
      settings: infoedit="Gateway out block.  Converts Xilinx fixed point inputs into ouputs of type Simulink integer, double, or fixed point.<P><P>Hardware notes:  In hardware these blocks become top level output ports or are discarded, depending on how they are configured."  xl_area=[0 0 0 0 1 0 0]  block_version=VER_STRING_GOES_HERE
  Phase I : Outport
  Phase Q : Outport  Port=2
  tag scope: PilotPhaseErr=global, preSpin_pilotCFOest=global, preSpin_pilotCFOest_valid=global, regRx_pilotCFOest=global, regRx_pilotCFOest_en=global
  wires:
  Pilot Phase Error Calc/2(Rst) -> Register2/2, CFO Calculation/3(Rst), Phase Noise Tracker/2(Reset)
  Delay12/1 -> Pilot Phase Error Calc/1(Raw I)
  Delay13/1 -> Pilot Phase Error Calc/2(Raw Q)
  Delay14/1 -> Pilot Phase Error Calc/4(Sym Index)
  I/1 -> Delay12/1
  Q/1 -> Delay13/1
  Xk_Index/1 -> Pilot Phase Error Calc/3(Xk_index)
  Sym_Index/1 -> Delay14/1
  Train/1 -> Pilot Phase Error Calc/9(Train)
  Register2/1 -> [PilotPhaseErr], Convert3/1
  HAA_I/1 -> Pilot Phase Error Calc/5(H_AA I)
  HAA_Q/1 -> Pilot Phase Error Calc/6(H_AA Q)
  HBA_I/1 -> Pilot Phase Error Calc/7(H_BA I)
  HBA_Q/1 -> Pilot Phase Error Calc/8(H_BA Q)
  Pilot Phase Error Calc/3(Vout) -> Register2/3, CFO Calculation/2(Vin), Phase Noise Tracker/3(Vin)
  Pilot Phase Error Calc/1(Phase Err) -> Register2/1, CFO Calculation/1(Phase Err), Phase Noise Tracker/1(Phase Err)
  Convert3/1 -> Rx_debug_PhaseError/1
  Phase Noise Tracker/1(Phases_I) -> Phase I/1
  Phase Noise Tracker/2(Phases_Q) -> Phase Q/1
  Pilot Phase Error Calc/4(" Sym Index") -> Phase Noise Tracker/4(Sym Index)
  CFO Calculation/1(CFO) -> [preSpin_pilotCFOest], Reinterpret/1
  CFO Calculation/2(Vout) -> [regRx_pilotCFOest_en], [preSpin_pilotCFOest_valid]
  Reinterpret/1 -> [regRx_pilotCFOest]
  Rst/1 -> Pilot Phase Error Calc/10("Rst ")

== system ofdm_txrx_supermimo_coded/OFDM Rx MIMO/FFT & Chan Est/Channel Estimation & Pilot Processing/Training? ==
  note: For SISO/multiplexing modes, numTrainingSymols is
      | doubled, as the FFT is run twice for each over-the-air
      | OFDM symbol period. For Alamouti mode, the FFT is
      | only run once per symbol, so numTrainingSymbols is
      | used as-is.
  note: The channel estimate buffers calculate the
      | running sum of estimates per subcarrier. In the first
      | training interval, the old estimates need to be ingored
      | so that the actual first estimate becomes the starting value
      | for the running sum. In SISO mode, the first training
      | interval lasts 2 FFTs (symIndex=[0 1]). In MIMO
      | mode, it lasts four (symIndex[0 1 2 3]).
  SynIndex : Inport
  Clear2 : xbsIndex_r4/Constant  const=1  gui_display_data_type=Fixed-point  arith_type=Unsigned  n_bits=1  bin_pt=0  preci_type=Single  exp_width=8  frac_width=24  explicit_period=off  period=1  equ=P=C  opselect=C  inp2=PCIN>>17  opr=+  inp1=P  carry=CIN  dbl_ovrd=off  block_type=constant
      settings: block_version=VER_STRING_GOES_HERE
  Concat : xbsIndex_r4/Concat  num_inputs=2  dbl_ovrd=off  block_type=concat
      settings: block_version=8.2.01
  Inverter1 : xbsIndex_r4/Inverter  en=off  latency=0  dbl_ovrd=off  block_type=inv
      settings: block_version=VER_STRING_GOES_HERE
  Inverter2 : xbsIndex_r4/Inverter  en=off  latency=0  dbl_ovrd=off  block_type=inv
      settings: block_version=VER_STRING_GOES_HERE
  Logical2 : xbsIndex_r4/Logical  logical_function=AND  inputs=2  en=off  latency=0  precision=Full  arith_type=Unsigned  n_bits=8  bin_pt=2  align_bp=on  dbl_ovrd=off  block_type=logical
      settings: xl_area=[1 0 0 1 0 0 0]  block_version=VER_STRING_GOES_HERE
  Mux : xbsIndex_r4/Mux  inputs=2  en=off  latency=0  precision=Full  arith_type=Unsigned  n_bits=16  bin_pt=14  quantization=Truncate  overflow=Wrap  dbl_ovrd=off  block_type=mux
  Relational : xbsIndex_r4/Relational  mode=a<=b  en=off  latency=0  dbl_ovrd=off  block_type=relational
      settings: xl_area=[0, 0, 0, 0, 0, 0, 0]  block_version=VER_STRING_GOES_HERE
  Relational1 : xbsIndex_r4/Relational  mode=a<b  en=off  latency=0  dbl_ovrd=off  block_type=relational
      settings: xl_area=[3 0 0 6 0 0 0]  block_version=VER_STRING_GOES_HERE
  Shift : xbsIndex_r4/Shift  shift_dir=Left  shift_bits=1  en=off  latency=0  precision=User Defined  arith_type=Unsigned  n_bits=1+ceil(log2(max_num_trainingSymbols))  bin_pt=0  quantization=Truncate  overflow=Flag as error  dbl_ovrd=off  block_type=shift
      settings: block_version=VER_STRING_GOES_HERE
  FirstSym : Outport
  Training : Outport  Port=2
  wires:
  [regRx_numTrainingSymbols] -> Shift/1, Mux/3
  SynIndex/1 -> Relational1/1, Relational/1
  Relational1/1 -> Training/1
  [regRx_AlamoutiMode] -> Mux/1
  Mux/1 -> Relational1/2
  Shift/1 -> Mux/2
  Logical2/1 -> Concat/1
  Clear2/1 -> Concat/2
  [regRx_SISOmode] -> Inverter1/1
  Concat/1 -> Relational/2
  Relational/1 -> FirstSym/1
  Inverter1/1 -> Logical2/2
  Inverter2/1 -> Logical2/1
  [regRx_AlamoutiMode] -> Inverter2/1
